simulink model "ofdm_txrx_supermimo"  (Simulink 7.1)
settings: Solver=VariableStepDiscrete  SolverMode=SingleTasking  StartTime=0.0  StopTime=simOnly_simLength  RelTol=1e-3
PostLoadFcn:
  | ofdm_rx_supermimo_init
InitFcn:
  | ofdm_rx_supermimo_init
StartFcn:
  | ofdm_rx_supermimo_init
values from neighbouring files (symbols this model uses):
  Action0_Config = 0* 31*2^18 + 0*2^24 + PHY_AUTORESPONSE_REQ_GOODHDR + PHY_AUTORESPONSE_REQ_GOODPKT + PHY_AUTORESPONSE_REQ_FLAGA    [ofdm_tx_supermimo_init.m]
  Action1_Config = 0* 62*2^18 + PHY_AUTORESPONSE_REQ_GOODHDR + PHY_AUTORESPONSE_REQ_GOODPKT    [ofdm_tx_supermimo_init.m]
  Action2_Config = 0    [ofdm_tx_supermimo_init.m]
  Action3_Config = 0    [ofdm_tx_supermimo_init.m]
  Action4_Config = 0    [ofdm_tx_supermimo_init.m]
  Action5_Config = 0    [ofdm_tx_supermimo_init.m]
  ActionID_AFTransmit = 31    [ofdm_tx_supermimo_init.m]
  ActionID_Disabled = 0    [ofdm_tx_supermimo_init.m]
  ActionID_SetFlagA = 62    [ofdm_tx_supermimo_init.m]
  ActionID_SetFlagB = 61    [ofdm_tx_supermimo_init.m]
  BER_RAM_init_size = 2048    [ofdm_tx_supermimo_init.m]
  CPLength = 16    [ofdm_tx_supermimo_init.m]
  longCorr_coef_i = [-4*real(LTS) 0]    [ofdm_rx_supermimo_init.m]
  longCorr_coef_q = [4*imag(LTS) 0]    [ofdm_rx_supermimo_init.m]
  Match0_Config = 0    [ofdm_tx_supermimo_init.m]
  Match1_Config = 0    [ofdm_tx_supermimo_init.m]
  Match2_Config = 0    [ofdm_tx_supermimo_init.m]
  Match3_Config = 0    [ofdm_tx_supermimo_init.m]
  Match4_Config = 0    [ofdm_tx_supermimo_init.m]
  Match5_Config = 0    [ofdm_tx_supermimo_init.m]
  Match6_Config = 0    [ofdm_tx_supermimo_init.m]
  Match7_Config = 0    [ofdm_tx_supermimo_init.m]
  max_CP_length = 16    [ofdm_tx_supermimo_init.m]
  max_num_baseRateSymbols = 31    [ofdm_tx_supermimo_init.m]
  max_num_subcarriers = 64    [ofdm_tx_supermimo_init.m]
  max_num_trainingSymbols = 15    [ofdm_tx_supermimo_init.m]
  max_numBytes = 16384    [ofdm_tx_supermimo_init.m]
  max_OFDM_symbols = 511    [ofdm_tx_supermimo_init.m]
  modConstellation_qam16 = .75*[1 3 -1 -3]./3    [ofdm_tx_supermimo_init.m]
  modConstellation_qam64 = 0.875*[3 1 5 7 -3 -1 -5 -7]./7    [ofdm_tx_supermimo_init.m]
  modConstellation_qpsk = [1 -1]./sqrt(2)    [ofdm_tx_supermimo_init.m]
  numBaseRateSymbols = ceil(numHeaderBytes / numBytes_BaseRateOFDMSymbol)    [ofdm_tx_supermimo_init.m]
  numSubcarriers = 64    [ofdm_tx_supermimo_init.m]
  numTrainingSymbols = 2    [ofdm_tx_supermimo_init.m]
  pilot_indicies = 7 + ( (64-21) * 2^8) + ( (64-7) * 2^16) + (21 * 2^24)    (= 356068103)    [ofdm_tx_supermimo_init.m]
  pilotValues = hex2dec('A57D') + (2^16 * hex2dec('5A82'))    [ofdm_tx_supermimo_init.m]
  pkt_crossCorr_thresh = 0.7    [ofdm_rx_supermimo_init.m]
  pkt_energy_thresh = 0    [ofdm_rx_supermimo_init.m]
  pktByteNums = numHeaderBytes +  				byteIndex_numPayloadBytes(1)*2^8 +  				byteIndex_numPayloadBytes(2)*2^16 +  				byteIndex_simpleDynModMasks * 2^24    [ofdm_rx_supermimo_init.m]
  PktDet_Delay = 58+32 +      Symbol_Timing_Offset*2^7 +      0*2^16 +      (117* 2^24)    (= 1962935642)    [ofdm_rx_supermimo_init.m]
  pktTiming_count_payloadStart = pktTiming_count_lsStart + pktTiming_ls_num*pktTiming_ls_length    [ofdm_rx_supermimo_init.m]
  preamble = preamble_scale*[shortsyms_10 longsyms_2]    [ofdm_tx_supermimo_init.m]
  preamble_ROM = preamble_I_ROM + preamble_Q_ROM*2^16    [ofdm_tx_supermimo_init.m]
  RAM_init_size = max_numBytes    (= 16384)    [ofdm_tx_supermimo_init.m]
  reg_AF_Tx_Blanking = (320+80) + ( (320+80+79)*2^16)    (= 31392144)    [ofdm_rx_supermimo_init.m]
  reg_AF_Tx_Scaling = 2944    [ofdm_rx_supermimo_init.m]
  reg_Interrupt_PktBuf_Control = reg_InterruptControl +  	1 * 2^16 +  	0 * 2^24    (= 65656)    [ofdm_rx_supermimo_init.m]
  reg_PilotCalcParams = (4 * 2^16) + (minPilotChanMag)    (= 262184.96)    [ofdm_rx_supermimo_init.m]
  regRx_chanEst_minMag = ((0.5)*2^15) + ((0.5 * 2^15)*2^16)    (= 1073758208)    [ofdm_rx_supermimo_init.m]
  regRx_coarseCalcCorrection = round((1.3e-3 - 1.2982e-3)*2^32)    [ofdm_rx_supermimo_init.m]
  regRx_pilotCalcCorrection = round(1.00115 * 2^31)    [ofdm_rx_supermimo_init.m]
  regRx_preCFOoptions = 0 * 2^0 +  	1 * 2^1 +  	0    (= 2)    [ofdm_rx_supermimo_init.m]
  rx_controlBits = 1 * 2  		 + 1 * 4  		 + 0 * 8  		 + 1 * 16 * rx_SISO_Mode  		 + 1 * 32  		 + 1 * 64  		 + 1 * 128  		 + 1 * 256  		 + 1 * 512  		 + 1 * 1024  		 + 1 * 4096  		 + 0 * 16384  		 + 0 * 32768  		 + 1 * 65536  		 + 0 * 131072  		 + 0 * 262144  		 + 1 * 524288  		 + 1 * 1048576 * tx_Alamouti_Mode  		 + 0 * 2097152           + 1 * 33554432  		 + 0 * 4194304  		 + 0 * 8388608  		 + 0 * 16777216  		 + 1 *  ...(+49 chars)    [ofdm_rx_supermimo_init.m]
  Rx_PktDet_LongCorr_Params = LongCorrCounterLoad + 0*2^8 + 0*2^16 + 255*2^24    (= 4278190325)    [ofdm_rx_supermimo_init.m]
  Rx_PktDet_LongCorrThresholds = 8000    [ofdm_rx_supermimo_init.m]
  rx_postEq_scaling = round(rxScaling*2^11) * (1 + 2^16)    [ofdm_rx_supermimo_init.m]
  RxDataScrambling_Seq = [40 198 78 63 82 173 102 245 48 111 172 115 147 230 216 93 72 65 62 2 205 242 122 90 128 83 105 97 73 10 5 252]    [ofdm_tx_supermimo_init.m]
  RxReg_FixedPktLen = 0    [ofdm_rx_supermimo_init.m]
  shortSymbol_time = shortSymbol_time(1:16).'    [ofdm_tx_supermimo_init.m]
  simOnly_numSamples = length(preamble)+( (numSubcarriers+CPLength)*(numTrainingSymbols + numBaseRateSymbols + numFullRateSymbols/2) )    [ofdm_tx_supermimo_init.m]
  train = [train train]    [ofdm_tx_supermimo_init.m]
  tx_controlBits = (antB_preambleShift * 2^4) + (2^1 * tx_Alamouti_Mode) +  	(2^0 * tx_SISO_Mode) + 4*0 + 8*1 + 0*256 + 512*0 + 1024*0 +  	0*hex2dec('1000') + 0*hex2dec('2000') + 0*hex2dec('4000') +  	1*hex2dec('8000') + 1*hex2dec('10000') + hex2dec('20000') +  	16*hex2dec('100000')    [ofdm_tx_supermimo_init.m]
  tx_delays = (50*2^0) + (0*2^8) + (30*2^12)    (= 122930)    [ofdm_tx_supermimo_init.m]
  tx_scaling = (3072) + (12288 * 2^16)    (= 805309440)    [ofdm_tx_supermimo_init.m]
  TxDataScrambling_Seq = [40 198 78 63 82 173 102 245 48 111 172 115 147 230 216 93 72 65 62 2 205 242 122 90 128 83 105 97 73 10 5 252]    [ofdm_tx_supermimo_init.m]
  txfilt_firpm = 0.95*txfilt_firpm./(max(txfilt_firpm))    [ofdm_tx_supermimo_init.m]
  txfilt_intfilt = [0 intfilt(4,4,.8)]    [ofdm_tx_supermimo_init.m]
  txReg_symbolCounts = (2^8 *numBaseRateSymbols ) + numTrainingSymbols    [ofdm_tx_supermimo_init.m]
  TxRx_FFTScaling = (3 * 2^0) +  	(2 * 2^2) +  	(1 * 2^4) +  	(1 * 2^6) +  	(1 * 2^8) +  	(0 * 2^10)    (= 347)    [ofdm_tx_supermimo_init.m]
undefined symbols (not defined in the file or in its folder): AntA, AntB, BaseRate, Down, gcbh, numBuffs, numBytes, Up, xlBlockLoadCallback, xlGetSimulinkPeriod, xlipgetpartsetting
libraries: xbsIndex_r4 x6035, simulink x4, dspsigops x4, commchan3 x2, dspsrcs4 x2, commrflib2 x2
(part 7 of 61)

== system ofdm_txrx_supermimo/OFDM Tx MIMO/OutputMuxes ==
  note: Cheap output-enable circuit. Output registers are
      | brought out of reset on the first 0->1 transition in the
      | LSB of Pream Index. In other words, the outputs are
      | enabled when the preamble starts, and disabled when
      | the entire packet is finished.
  Pream Index : Inport  OutDataType=sfix(16)  OutScaling=2^0
  AntA I : Inport  Port=2  OutDataType=sfix(16)  OutScaling=2^0
  AntA Q : Inport  Port=3  OutDataType=sfix(16)  OutScaling=2^0
  AntB I : Inport  Port=4  OutDataType=sfix(16)  OutScaling=2^0
  AntB Q : Inport  Port=5  OutDataType=sfix(16)  OutScaling=2^0
  vin : Inport  Port=6  OutDataType=sfix(16)  OutScaling=2^0
  Pkt Done : Inport  Port=7  OutDataType=sfix(16)  OutScaling=2^0
  Antenna Swapping : Subsystem
  Constant1 : xbsIndex_r4/Constant  arith_type=Boolean  const=0  n_bits=1  bin_pt=0  explicit_period=off  period=2  equ=P=C  opselect=C  inp2=PCIN>>17  opr=+  inp1=P  carry=CIN  dbl_ovrd=off  block_type=constant
      settings: block_version=VER_STRING_GOES_HERE
  Constant7 : xbsIndex_r4/Constant  arith_type=Boolean  const=1  n_bits=1  bin_pt=0  explicit_period=off  period=2  equ=P=C  opselect=C  inp2=PCIN>>17  opr=+  inp1=P  carry=CIN  dbl_ovrd=off  block_type=constant
      settings: block_version=VER_STRING_GOES_HERE
  Delay1 : xbsIndex_r4/Delay  en=off  latency=1  dbl_ovrd=off  reg_retiming=off  block_type=delay
      settings: infoedit=Hardware notes: A delay line is a chain, each link of which is an SRL16 followed by a flip-flop. If register retiming is enabled, the delay line is a chain of flip-flops.  block_version=VER_STRING_GOES_HERE
  Delay2 : xbsIndex_r4/Delay  en=off  latency=1  dbl_ovrd=off  reg_retiming=off  block_type=delay
      settings: infoedit=Hardware notes: A delay line is a chain, each link of which is an SRL16 followed by a flip-flop. If register retiming is enabled, the delay line is a chain of flip-flops.  block_version=VER_STRING_GOES_HERE
  Inverter : xbsIndex_r4/Inverter  en=off  latency=0  dbl_ovrd=off  block_type=inv
      settings: xl_area=[0, 0, 0, 0, 0, 0, 0]  block_version=VER_STRING_GOES_HERE
  LSB : xbsIndex_r4/Slice  nbits=1  boolean_output=on  mode=Lower Bit Location + Width  bit1=0  base1=MSB of Input  bit0=0  base0=LSB of Input  dbl_ovrd=off  block_type=slice
      settings: infoedit="Extracts a given range of bits from each input sample and presents it at the output.  The output type is ordinarily unsigned with binary point at zero, but can be Boolean when the slice is one bit wide.<P><P>Hardware notes: In hardware this block costs nothing."  block_version=VER_STRING_GOES_HERE
  Logical1 : xbsIndex_r4/Logical  logical_function=OR  inputs=2  en=off  latency=0  precision=Full  arith_type=Unsigned  n_bits=8  bin_pt=2  align_bp=on  dbl_ovrd=off  block_type=logical
      settings: xl_area=[1 0 0 1 0 0 0]  block_version=VER_STRING_GOES_HERE
  Logical2 : xbsIndex_r4/Logical  logical_function=OR  inputs=2  en=off  latency=0  precision=Full  arith_type=Unsigned  n_bits=8  bin_pt=2  align_bp=on  dbl_ovrd=off  block_type=logical
      settings: xl_area=[1 0 0 1 0 0 0]  block_version=VER_STRING_GOES_HERE
  Logical3 : xbsIndex_r4/Logical  logical_function=OR  inputs=2  en=off  latency=0  precision=Full  arith_type=Unsigned  n_bits=8  bin_pt=2  align_bp=on  dbl_ovrd=off  block_type=logical
      settings: xl_area=[1 0 0 1 0 0 0]  block_version=VER_STRING_GOES_HERE
  Mux{,1..3} (4 alike) : xbsIndex_r4/Mux  inputs=2  en=off  latency=0  precision=User Defined  arith_type="Signed  (2's comp)"  n_bits=14  bin_pt=13  quantization=Truncate  overflow=Wrap  dbl_ovrd=off  block_type=mux
      settings: xl_area=[7 0 0 14 0 0 0]  block_version=VER_STRING_GOES_HERE
  Payload Running : xbsIndex_r4/Register  init=0  rst=on  en=on  dbl_ovrd=off  block_type=register
      settings: xl_area=[1 1 0 0 0 0 0]  block_version=VER_STRING_GOES_HERE
  Payload Running1 : xbsIndex_r4/Register  init=1  rst=on  en=on  dbl_ovrd=off  block_type=register
      settings: xl_area=[1 1 0 0 0 0 0]  block_version=VER_STRING_GOES_HERE
  Posedge2 : Subsystem
  Preamble Generation : Subsystem
  Preambles : Scope  NumInputPorts=7
      settings: TimeRange=9328  YMin=-0.2~-0.25~-1~-1~0~0~0  YMax=0.15~0.15~1~1~1~3500~1  SaveName=ScopeData13
  Register{,1..3} (4 alike) : xbsIndex_r4/Register  init=0  rst=on  en=off  dbl_ovrd=off  block_type=register
      settings: xl_area=[0, 0, 0, 0, 0, 0, 0]  block_version=VER_STRING_GOES_HERE
  TxDone Logic : Subsystem
  TxRunning Outputs : Subsystem
  done1 : xbsIndex_r4/Gateway Out  hdl_port=off  timing_constraint=None  locs_specified=off  LOCs={}  block_type=gatewayout
      settings: infoedit="Gateway out block.  Converts Xilinx fixed point inputs into ouputs of type Simulink integer, double, or fixed point.<P><P>Hardware notes:  In hardware these blocks become top level output ports or are discarded, depending on how they are configured."  xl_area=[0 0 0 0 1 0 0]  block_version=VER_STRING_GOES_HERE
  done4 : xbsIndex_r4/Gateway Out  hdl_port=off  timing_constraint=None  locs_specified=off  LOCs={}  block_type=gatewayout
      settings: infoedit="Gateway out block.  Converts Xilinx fixed point inputs into ouputs of type Simulink integer, double, or fixed point.<P><P>Hardware notes:  In hardware these blocks become top level output ports or are discarded, depending on how they are configured."  xl_area=[0 0 0 0 1 0 0]  block_version=VER_STRING_GOES_HERE
  done5 : xbsIndex_r4/Gateway Out  hdl_port=off  timing_constraint=None  locs_specified=off  LOCs={}  block_type=gatewayout
      settings: infoedit="Gateway out block.  Converts Xilinx fixed point inputs into ouputs of type Simulink integer, double, or fixed point.<P><P>Hardware notes:  In hardware these blocks become top level output ports or are discarded, depending on how they are configured."  xl_area=[0 0 0 0 1 0 0]  block_version=VER_STRING_GOES_HERE
  Payload : Outport  OutDataType=sfix(16)  OutScaling=2^0
  " AntA I" : Outport  Port=2  OutDataType=sfix(16)  OutScaling=2^0
  " AntA Q" : Outport  Port=3  OutDataType=sfix(16)  OutScaling=2^0
  " AntB I" : Outport  Port=4  OutDataType=sfix(16)  OutScaling=2^0
  " AntB Q" : Outport  Port=5  OutDataType=sfix(16)  OutScaling=2^0
  tag scope: Tx_Running=global
  wires:
  Logical1/1 -> Mux/1, Mux1/1, Mux2/1, Mux3/1, Logical3/1
  Constant7/1 -> Payload Running/1
  TxDone Logic/1(Done) -> Payload Running/2, Delay1/1
  vin/1 -> Payload Running/3, Logical1/2
  AntA I/1 -> Mux/3
  AntA Q/1 -> Mux1/3
  Constant1/1 -> Payload Running1/1
  Payload Running1/1 -> Antenna Swapping/1(Rst), Register/2, Register1/2, Register2/2, Register3/2, Inverter/1
  LSB/1 -> Posedge2/1(D)
  Logical2/1 -> LSB/1, Antenna Swapping/2(PreamIndex), Preamble Generation/1(SampInd)
  Preamble Generation/2(AntA_Q) -> Mux1/2
  Register/1 -> " AntA I"/1
  Register1/1 -> " AntA Q"/1
  Mux2/1 -> Antenna Swapping/5(AntB_I)
  Mux3/1 -> Antenna Swapping/6(AntB_Q)
  Register2/1 -> " AntB I"/1
  Register3/1 -> " AntB Q"/1
  AntB I/1 -> Mux2/3
  AntB Q/1 -> Mux3/3
  Preamble Generation/3(AntB_I) -> Mux2/2
  Preamble Generation/4(AntB_Q) -> Mux3/2
  Payload Running/1 -> Logical1/1
  Delay1/1 -> Payload Running1/2
  Delay2/1 -> Payload/1
  Preamble Generation/1(AntA_I) -> Mux/2, done1/1
  Mux/1 -> Antenna Swapping/3(AntA_I)
  Mux1/1 -> Antenna Swapping/4(AntA_Q)
  Antenna Swapping/2(" AntA_I") -> Register/1
  Antenna Swapping/3(" AntA_Q") -> Register1/1
  Antenna Swapping/4(" AntB_I") -> Register2/1
  Antenna Swapping/5(" AntB_Q") -> Register3/1
  Pream Index/1 -> Logical2/2, done5/1
  [AF_PreambleIndex] -> Logical2/1, done4/1
  Posedge2/1(Q) -> Payload Running1/3
  Pkt Done/1 -> TxDone Logic/1(Pkt Done)
  Logical3/1 -> Delay2/1
  Antenna Swapping/1(AF Payload) -> Logical3/2
  Inverter/1 -> [Tx_Running], TxRunning Outputs/1(Tx)
  done4/1 -> Preambles/1
  done5/1 -> Preambles/2
  done1/1 -> Preambles/3

== system ofdm_txrx_supermimo/OFDM Tx MIMO/Pre-Spin,  Filters & DACs ==
  note: Delay for mults (2)
      | and filters (6)
  Payload : Inport  OutDataType=sfix(16)  OutScaling=2^0
  A_I : Inport  Port=2  OutDataType=sfix(16)  OutScaling=2^0
  A_Q : Inport  Port=3  OutDataType=sfix(16)  OutScaling=2^0
  B_I : Inport  Port=4  OutDataType=sfix(16)  OutScaling=2^0
  B_Q : Inport  Port=5  OutDataType=sfix(16)  OutScaling=2^0
  A*conj(B) : Subsystem
  "A*conj(B) " : Subsystem
  Constant1 : xbsIndex_r4/Constant  arith_type=Signed (2's comp)  const=0  n_bits=16  bin_pt=15  explicit_period=on  period=4  equ=P=C  opselect=C  inp2=PCIN>>17  opr=+  inp1=P  carry=CIN  dbl_ovrd=off  block_type=constant
      settings: block_version=VER_STRING_GOES_HERE
  Constant2 : xbsIndex_r4/Constant  arith_type=Signed (2's comp)  const=1  n_bits=16  bin_pt=15  explicit_period=on  period=4  equ=P=C  opselect=C  inp2=PCIN>>17  opr=+  inp1=P  carry=CIN  dbl_ovrd=off  block_type=constant
      settings: block_version=VER_STRING_GOES_HERE
  Convert1 : xbsIndex_r4/Convert  arith_type=Unsigned  n_bits=1  bin_pt=0  quantization=Truncate  overflow=Wrap  latency=0  dbl_ovrd=off  pipeline=off  block_type=convert
      settings: xl_area=[0, 0, 0, 0, 0, 0, 0]  block_version=VER_STRING_GOES_HERE
  Delay1 : xbsIndex_r4/Delay  en=off  latency=8  dbl_ovrd=off  reg_retiming=off  block_type=delay
  Down Sample : xbsIndex_r4/Down Sample  sample_ratio=2  sample_phase="Last Value of Frame  (most efficient)"  en=off  latency=1  dbl_ovrd=off  block_type=dsamp
      settings: block_version=VER_STRING_GOES_HERE
  Filters : Subsystem
  Logical1 : xbsIndex_r4/Logical  logical_function=OR  inputs=2  en=off  latency=0  precision=Full  arith_type=Unsigned  n_bits=8  bin_pt=2  align_bp=on  dbl_ovrd=off  block_type=logical
      settings: xl_area=[1 0 0 1 0 0 0]  block_version=VER_STRING_GOES_HERE
  Mult{1..3,7} (4 alike) : xbsIndex_r4/Mult  precision=User Defined  arith_type="Signed  (2's comp)"  n_bits=16  bin_pt=15  quantization=Truncate  overflow=Saturate  en=off  latency=1  dbl_ovrd=off  use_behavioral_HDL=off  use_embedded=on  opt=Speed  optimum_pipeline=off  pipeline=on  use_rpm=on  placement_style=Triangular  block_type=mult
      settings: infoedit=Hardware notes: To use the internal pipeline stage of the dedicated multiplier you must select 'Pipeline for maximum performance'.  block_version=10.1.3
  Mux1 : xbsIndex_r4/Mux  inputs=2  en=off  latency=0  precision=Full  arith_type=Unsigned  n_bits=16  bin_pt=14  quantization=Truncate  overflow=Wrap  dbl_ovrd=off  block_type=mux
      settings: block_version=VER_STRING_GOES_HERE
  Mux3 : xbsIndex_r4/Mux  inputs=2  en=off  latency=0  precision=Full  arith_type=Unsigned  n_bits=16  bin_pt=14  quantization=Truncate  overflow=Wrap  dbl_ovrd=off  block_type=mux
      settings: block_version=VER_STRING_GOES_HERE
  Mux4 : xbsIndex_r4/Mux  inputs=2  en=off  latency=0  precision=Full  arith_type=Unsigned  n_bits=16  bin_pt=14  quantization=Truncate  overflow=Wrap  dbl_ovrd=off  block_type=mux
      settings: block_version=VER_STRING_GOES_HERE
  Tx : Scope  NumInputPorts=7
      settings: TimeRange=9328  YMin=-1~-1~-1~-1~-0.4~-0.4~0.25  YMax=1~1~1~1~0.4~0.5~1.5  SaveName=ScopeData9
  Tx_AntA_DAC_I : xbsIndex_r4/Gateway Out  hdl_port=on  timing_constraint=None  locs_specified=off  LOCs={}  block_type=gatewayout
      settings: infoedit="Gateway out block.  Converts Xilinx fixed point inputs into ouputs of type Simulink integer, double, or fixed point.<P><P>Hardware notes:  In hardware these blocks become top level output ports or are discarded, depending on how they are configured."  block_version=10.1.3
  Tx_AntA_DAC_Q : xbsIndex_r4/Gateway Out  hdl_port=on  timing_constraint=None  locs_specified=off  LOCs={}  block_type=gatewayout
      settings: infoedit="Gateway out block.  Converts Xilinx fixed point inputs into ouputs of type Simulink integer, double, or fixed point.<P><P>Hardware notes:  In hardware these blocks become top level output ports or are discarded, depending on how they are configured."  block_version=10.1.3
  Tx_AntB_DAC_I : xbsIndex_r4/Gateway Out  hdl_port=on  timing_constraint=None  locs_specified=off  LOCs={}  block_type=gatewayout
      settings: infoedit="Gateway out block.  Converts Xilinx fixed point inputs into ouputs of type Simulink integer, double, or fixed point.<P><P>Hardware notes:  In hardware these blocks become top level output ports or are discarded, depending on how they are configured."  block_version=10.1.3
  Tx_AntB_DAC_Q : xbsIndex_r4/Gateway Out  hdl_port=on  timing_constraint=None  locs_specified=off  LOCs={}  block_type=gatewayout
      settings: infoedit="Gateway out block.  Converts Xilinx fixed point inputs into ouputs of type Simulink integer, double, or fixed point.<P><P>Hardware notes:  In hardware these blocks become top level output ports or are discarded, depending on how they are configured."  block_version=10.1.3
  Up Sample13 : xbsIndex_r4/Up Sample  sample_ratio=2  copy_samples=on  dbl_ovrd=off  block_type=usamp
      settings: infoedit="Up samples input data.  Inserted values  can be zeros or copies of the most recent input sample.<P><P>Hardware notes: No hardware is needed if inserted values are copies of the input sample; otherwise, a mux and single bit flip-flop are used."  block_version=10.1.3
  done{1..7} (7 alike) : xbsIndex_r4/Gateway Out  hdl_port=off  timing_constraint=None  locs_specified=off  LOCs={}  block_type=gatewayout
      settings: infoedit="Gateway out block.  Converts Xilinx fixed point inputs into ouputs of type Simulink integer, double, or fixed point.<P><P>Hardware notes:  In hardware these blocks become top level output ports or are discarded, depending on how they are configured."  xl_area=[0 0 0 0 1 0 0]  block_version=VER_STRING_GOES_HERE
  " A_I" : Outport  OutDataType=sfix(16)  OutScaling=2^0
  " A_Q" : Outport  Port=2  OutDataType=sfix(16)  OutScaling=2^0
  " B_I" : Outport  Port=3  OutDataType=sfix(16)  OutScaling=2^0
  " B_Q" : Outport  Port=4  OutDataType=sfix(16)  OutScaling=2^0
  wires:
  Filters/1(" I_A") -> Mult7/2, done3/1
  Tx_AntA_DAC_I/1 -> " A_I"/1
  Filters/2(" Q_A") -> Mult1/2, done6/1
  Tx_AntA_DAC_Q/1 -> " A_Q"/1
  Tx_AntB_DAC_I/1 -> " B_I"/1
  Tx_AntB_DAC_Q/1 -> " B_Q"/1
  Filters/4(" Q_B") -> Mult3/2
  Payload/1 -> Delay1/1
  [TxReg_PreambleScaling] -> Mux4/2
  [TxReg_postIFFTScaling] -> Mux4/3
  Mult1/1 -> Tx_AntA_DAC_Q/1, done1/1
  Mux4/1 -> Mult7/1, Mult1/1, Mult2/1, Mult3/1, done7/1
  Up Sample13/1 -> Mux4/1
  Filters/3(" I_B") -> Mult2/2
  Mult3/1 -> Tx_AntB_DAC_Q/1
  Mult2/1 -> Tx_AntB_DAC_I/1
  B_Q/1 -> "A*conj(B) "/4(Im[A])
  B_I/1 -> "A*conj(B) "/3(Re[A])
  [RxAutoReplyProc_UsePreCFO] -> Convert1/1
  [CFO_DDSOut_I] -> Mux1/3
  [CFO_DDSOut_Q] -> Mux3/3
  Down Sample/1 -> Mux3/1, Mux1/1
  Constant2/1 -> Mux1/2
  Constant1/1 -> Mux3/2
  Logical1/1 -> Down Sample/1
  [regTx_AlwaysUsePreSpin] -> Logical1/1
  Convert1/1 -> Logical1/2
  Delay1/1 -> Up Sample13/1
  Mux1/1 -> A*conj(B)/4(Re[B]), "A*conj(B) "/1(Re[B])
  Mux3/1 -> A*conj(B)/3(Im[B]), "A*conj(B) "/2(Im[B])
  A_I/1 -> A*conj(B)/1(Re[A])
  A_Q/1 -> A*conj(B)/2(Im[A])
  Mult7/1 -> Tx_AntA_DAC_I/1, done2/1
  done4/1 -> Tx/1
  done5/1 -> Tx/2
  done3/1 -> Tx/3
  done6/1 -> Tx/4
  done2/1 -> Tx/5
  done1/1 -> Tx/6
  done7/1 -> Tx/7
  A*conj(B)/1(Re) -> Filters/1(I_A), done4/1
  A*conj(B)/2(Im) -> Filters/2(Q_A), done5/1
  "A*conj(B) "/1(Re) -> Filters/3(I_B)
  "A*conj(B) "/2(Im) -> Filters/4(Q_B)

== system ofdm_txrx_supermimo/OFDM Tx MIMO/Training_Data ==
  CPn_Data : Inport  OutDataType=sfix(16)  OutScaling=2^0
  train_pay : Inport  Port=2  OutDataType=sfix(16)  OutScaling=2^0
  BaseRate_En : Inport  Port=3  OutDataType=sfix(16)  OutScaling=2^0
  FFT En : Inport  Port=4  OutDataType=sfix(16)  OutScaling=2^0
  Start : Inport  Port=5  OutDataType=sfix(16)  OutScaling=2^0
  packet_done : Inport  Port=6  OutDataType=sfix(16)  OutScaling=2^0
  xn_index : Inport  Port=7  OutDataType=sfix(16)  OutScaling=2^0
  Ant Selector : Subsystem
  Convert2 : xbsIndex_r4/Convert  arith_type="Signed  (2's comp)"  n_bits=16  bin_pt=15  quantization=Truncate  overflow=Wrap  latency=0  dbl_ovrd=off  pipeline=off  block_type=convert
      settings: xl_area=[0 0 0 0 0 0 0]  block_version=VER_STRING_GOES_HERE
  Convert3 : xbsIndex_r4/Convert  arith_type="Signed  (2's comp)"  n_bits=16  bin_pt=15  quantization=Truncate  overflow=Wrap  latency=0  dbl_ovrd=off  pipeline=off  block_type=convert
      settings: xl_area=[0 0 0 0 0 0 0]  block_version=VER_STRING_GOES_HERE
  Delay2 : xbsIndex_r4/Delay  en=off  latency=1  dbl_ovrd=off  reg_retiming=off  block_type=delay
      settings: infoedit=Hardware notes: A delay line is a chain, each link of which is an SRL16 followed by a flip-flop. If register retiming is enabled, the delay line is a chain of flip-flops.  xl_area=[1 1 0 0 0 0 0]  block_version=VER_STRING_GOES_HERE
  FlexibleMod : Subsystem
  Logical : xbsIndex_r4/Logical  logical_function=AND  inputs=3  en=off  latency=0  precision=Full  arith_type=Unsigned  n_bits=8  bin_pt=2  align_bp=on  dbl_ovrd=off  block_type=logical
      settings: xl_area=[0, 0, 0, 0, 0, 0, 0]  block_version=VER_STRING_GOES_HERE
  Logical1 : xbsIndex_r4/Logical  logical_function=OR  inputs=2  en=off  latency=0  precision=Full  arith_type=Unsigned  n_bits=8  bin_pt=2  align_bp=on  dbl_ovrd=off  block_type=logical
      settings: xl_area=[0, 0, 0, 0, 0, 0, 0]  block_version=VER_STRING_GOES_HERE
  Logical5 : xbsIndex_r4/Logical  logical_function=AND  inputs=2  en=off  latency=0  precision=Full  arith_type=Unsigned  n_bits=8  bin_pt=2  align_bp=on  dbl_ovrd=off  block_type=logical
      settings: xl_area=[0, 0, 0, 0, 0, 0, 0]  block_version=VER_STRING_GOES_HERE
  Training_Pilots : Subsystem
  tx_Q : Outport  OutDataType=sfix(16)  OutScaling=2^0
  tx_I : Outport  Port=2  OutDataType=sfix(16)  OutScaling=2^0
  Payload Done : Outport  Port=3  OutDataType=sfix(16)  OutScaling=2^0
  wires:
  FlexibleMod/2(Q) -> Training_Pilots/3(Q_mod)
  FlexibleMod/1(I) -> Training_Pilots/2(I_mod)
  packet_done/1 -> FlexibleMod/2(packet_done), Logical1/1
  xn_index/1 -> FlexibleMod/1(ifft_index), Training_Pilots/1(Xn_index)
  train_pay/1 -> Delay2/1
  Logical/1 -> FlexibleMod/3(vin)
  Start/1 -> FlexibleMod/4(start)
  BaseRate_En/1 -> Ant Selector/1(BaseRate), Logical5/2
  CPn_Data/1 -> Ant Selector/2(CP#_Data), Logical/2
  Delay2/1 -> Logical5/1, Training_Pilots/4(train#), Logical/3
  Logical5/1 -> FlexibleMod/5(BaseRate_En)
  Ant Selector/1(Sel) -> FlexibleMod/6(ant_sel), Training_Pilots/5(antSel)
  FFT En/1 -> Logical/1
  Training_Pilots/1(tx_I) -> Convert3/1
  Training_Pilots/2(tx_Q) -> Convert2/1
  Convert3/1 -> tx_I/1
  Convert2/1 -> tx_Q/1
  Logical1/1 -> Training_Pilots/6(rst), Ant Selector/3(Rst)
  [TxReset] -> Logical1/2
  FlexibleMod/3(Payload Done) -> Payload Done/1

== system ofdm_txrx_supermimo/OFDM Tx MIMO/Tx Reset Logic ==
  Constant3 : Constant  Value=0  OutDataType=sfix(16)  OutScaling=2^0
  Logical : xbsIndex_r4/Logical  logical_function=OR  inputs=2  en=off  latency=0  precision=Full  arith_type=Unsigned  n_bits=16  bin_pt=0  align_bp=on  dbl_ovrd=off  block_type=logical
      settings: block_version=VER_STRING_GOES_HERE
  Pulse Generator1 : DiscretePulseGenerator  Period=1e6  PulseWidth=4  PhaseDelay=2750
  ToBool : xbsIndex_r4/Convert  arith_type=Boolean  n_bits=16  bin_pt=14  quantization=Truncate  overflow=Wrap  latency=0  dbl_ovrd=off  pipeline=off  block_type=convert
      settings: block_version=VER_STRING_GOES_HERE
  Tx_Reset : xbsIndex_r4/Gateway In  arith_type=Unsigned  n_bits=1  bin_pt=0  quantization="Round  (unbiased: +/- Inf)"  overflow=Saturate  period=4  dbl_ovrd=off  timing_constraint=None  locs_specified=off  LOCs={}  block_type=gatewayin
      settings: infoedit="Gateway in block.  Converts inputs of type Simulink integer, double and fixed point to  Xilinx fixed point type.<P><P>Hardware notes:  In hardware these blocks become top level input ports."  xl_area=[0, 0, 0, 0, 0, 0, 0]  block_version=VER_STRING_GOES_HERE
  tag scope: TxReset=global
  wires:
  Tx_Reset/1 -> Logical/1
  Logical/1 -> ToBool/1
  ToBool/1 -> [TxReset]
  Constant3/1 -> Tx_Reset/1
  [TxReg_TxReset] -> Logical/2

== system ofdm_txrx_supermimo/OFDM Tx MIMO/TxControl ==
  note: 0=Waiting for Cyclic Prefix
      | 1=Processing real data
  note: 0=Sending training symbols
      | 1=Processing real data
  note: 1 cycle delay to align CPn_data
      | with IFFT start. FFT core begins
      | accepting data 1 cycle after
      | rising edge of start.
  note: Three inputs to AND for IFFT start:
      | 1) Start when first data sample is ready
      | 2) Start when samp/sym counters are enabled
      | (i.e. during payload, not training)
      | 3) Not after last symbol is finished
      | (i.e. when coutners are being reset)
  StartTx : Inport  OutDataType=sfix(16)  OutScaling=2^0
  PktDone : Inport  Port=2  OutDataType=sfix(16)  OutScaling=2^0
  16_8LSB : xbsIndex_r4/Slice  nbits=ceil(log2(max_num_baseRateSymbols+1))  boolean_output=off  mode=Lower Bit Location + Width  bit1=0  base1=MSB of Input  bit0=8  base0=LSB of Input  dbl_ovrd=off  block_type=slice
      settings: infoedit="Extracts a given range of bits from each input sample and presents it at the output.  The output type is ordinarily unsigned with binary point at zero, but can be Boolean when the slice is one bit wide.<P><P>Hardware notes: In hardware this block costs nothing."  block_version=VER_STRING_GOES_HERE
  2x : Subsystem
  2x1 : Subsystem
  8LSB : xbsIndex_r4/Slice  nbits=ceil(log2(max_num_trainingSymbols+1))  boolean_output=off  mode=Lower Bit Location + Width  bit1=0  base1=MSB of Input  bit0=0  base0=LSB of Input  dbl_ovrd=off  block_type=slice
      settings: infoedit="Extracts a given range of bits from each input sample and presents it at the output.  The output type is ordinarily unsigned with binary point at zero, but can be Boolean when the slice is one bit wide.<P><P>Hardware notes: In hardware this block costs nothing."  block_version=VER_STRING_GOES_HERE
  AddSub1 : xbsIndex_r4/AddSub  mode=Addition  use_carryin=off  use_carryout=off  en=off  latency=0  precision=Full  arith_type=Unsigned  n_bits=10  bin_pt=0  quantization=Truncate  overflow=Wrap  dbl_ovrd=off  use_behavioral_HDL=on  pipelined=off  use_rpm=on  hw_selection=Fabric  block_type=addsub
      settings: xl_area=[0, 0, 0, 0, 0, 0, 0]  block_version=VER_STRING_GOES_HERE
  Constant1 : xbsIndex_r4/Constant  Orientation=left  arith_type=Unsigned  const=length(preamble)-148  n_bits=ceil(log2(length(preamble)))  bin_pt=0  explicit_period=off  period=1  equ=P=C  opselect=C  inp2=PCIN>>17  opr=+  inp1=P  carry=CIN  dbl_ovrd=off  block_type=constant
      settings: block_version=VER_STRING_GOES_HERE
  Constant2 : xbsIndex_r4/Constant  arith_type=Boolean  const=1  n_bits=1  bin_pt=0  explicit_period=off  period=1  equ=P=C  opselect=C  inp2=PCIN>>17  opr=+  inp1=P  carry=CIN  dbl_ovrd=off  block_type=constant
      settings: block_version=VER_STRING_GOES_HERE
  Constant3 : xbsIndex_r4/Constant  Orientation=left  arith_type=Unsigned  const=length(preamble)-1  n_bits=ceil(log2(length(preamble)))  bin_pt=0  explicit_period=off  period=1  equ=P=C  opselect=C  inp2=PCIN>>17  opr=+  inp1=P  carry=CIN  dbl_ovrd=off  block_type=constant
      settings: block_version=VER_STRING_GOES_HERE
  Constant4 : xbsIndex_r4/Constant  Orientation=left  arith_type=Unsigned  const=numSubcarriers+CPLength-1  n_bits=ceil(log2(max_num_subcarriers+CPLength+1))  bin_pt=0  explicit_period=off  period=1  equ=P=C  opselect=C  inp2=PCIN>>17  opr=+  inp1=P  carry=CIN  dbl_ovrd=off  block_type=constant
      settings: block_version=VER_STRING_GOES_HERE
  Constant5 : xbsIndex_r4/Constant  arith_type=Boolean  const=1  n_bits=1  bin_pt=0  explicit_period=off  period=1  equ=P=C  opselect=C  inp2=PCIN>>17  opr=+  inp1=P  carry=CIN  dbl_ovrd=off  block_type=constant
      settings: block_version=VER_STRING_GOES_HERE
  Constant6 : xbsIndex_r4/Constant  arith_type=Boolean  const=1  n_bits=1  bin_pt=0  explicit_period=off  period=1  equ=P=C  opselect=C  inp2=PCIN>>17  opr=+  inp1=P  carry=CIN  dbl_ovrd=off  block_type=constant
      settings: AttributesFormatString=%<explicit_period>  block_version=VER_STRING_GOES_HERE
  Constant7 : xbsIndex_r4/Constant  arith_type=Unsigned  const=numSubcarriers-1  n_bits=log2(numSubcarriers)  bin_pt=0  explicit_period=off  period=1  equ=P=C  opselect=C  inp2=PCIN>>17  opr=+  inp1=P  carry=CIN  dbl_ovrd=off  block_type=constant
      settings: block_version=VER_STRING_GOES_HERE
  Constant8 : xbsIndex_r4/Constant  Orientation=left  arith_type=Unsigned  const=0  n_bits=1  bin_pt=0  explicit_period=off  period=1  equ=P=C  opselect=C  inp2=PCIN>>17  opr=+  inp1=P  carry=CIN  dbl_ovrd=off  block_type=constant
      settings: block_version=VER_STRING_GOES_HERE
  Convert : xbsIndex_r4/Convert  arith_type=Boolean  n_bits=8  bin_pt=6  quantization=Truncate  overflow=Wrap  latency=0  dbl_ovrd=off  pipeline=off  block_type=convert
      settings: xl_area=[0, 0, 0, 0, 0, 0, 0]  block_version=VER_STRING_GOES_HERE
  Delay : xbsIndex_r4/Delay  en=off  latency=1  dbl_ovrd=off  reg_retiming=off  block_type=delay
      settings: infoedit=Hardware notes: A delay line is a chain, each link of which is an SRL16 followed by a flip-flop. If register retiming is enabled, the delay line is a chain of flip-flops.  xl_area=[1 1 0 0 0 0 0]  block_version=VER_STRING_GOES_HERE
  Delay1 : xbsIndex_r4/Delay  en=off  latency=1  dbl_ovrd=off  reg_retiming=off  block_type=delay
      settings: infoedit=Hardware notes: A delay line is a chain, each link of which is an SRL16 followed by a flip-flop. If register retiming is enabled, the delay line is a chain of flip-flops.  xl_area=[1 1 0 0 0 0 0]  block_version=VER_STRING_GOES_HERE
  Down Sample : xbsIndex_r4/Down Sample  sample_ratio=2  sample_phase=First Value of Frame  en=off  latency=0  dbl_ovrd=off  block_type=dsamp
      settings: block_version=VER_STRING_GOES_HERE
  Down Sample1 : xbsIndex_r4/Down Sample  sample_ratio=2  sample_phase=First Value of Frame  en=off  latency=0  dbl_ovrd=off  block_type=dsamp
      settings: block_version=VER_STRING_GOES_HERE
  Down Sample4 : xbsIndex_r4/Down Sample  sample_ratio=2  sample_phase="Last Value of Frame  (most efficient)"  en=off  latency=1  dbl_ovrd=off  block_type=dsamp
      settings: block_version=VER_STRING_GOES_HERE
  FFT Start Gen : Subsystem  Orientation=left
  Logical1 : xbsIndex_r4/Logical  logical_function=OR  inputs=2  en=off  latency=0  precision=Full  arith_type=Unsigned  n_bits=8  bin_pt=2  align_bp=on  dbl_ovrd=off  block_type=logical
      settings: xl_area=[1 0 0 1 0 0 0]  block_version=VER_STRING_GOES_HERE
  Logical2 : xbsIndex_r4/Logical  logical_function=OR  inputs=3  en=off  latency=0  precision=Full  arith_type=Unsigned  n_bits=8  bin_pt=2  align_bp=on  dbl_ovrd=off  block_type=logical
      settings: xl_area=[1 0 0 1 0 0 0]  block_version=VER_STRING_GOES_HERE
  Logical4 : xbsIndex_r4/Logical  logical_function=OR  inputs=2  en=off  latency=0  precision=Full  arith_type=Unsigned  n_bits=8  bin_pt=2  align_bp=on  dbl_ovrd=off  block_type=logical
      settings: xl_area=[1 0 0 1 0 0 0]  block_version=VER_STRING_GOES_HERE
  Logical6 : xbsIndex_r4/Logical  logical_function=AND  inputs=3  en=off  latency=0  precision=Full  arith_type=Unsigned  n_bits=1  bin_pt=0  align_bp=on  dbl_ovrd=off  block_type=logical
      settings: xl_area=[1 0 0 1 0 0 0]  block_version=VER_STRING_GOES_HERE
  Logical7 : xbsIndex_r4/Logical  logical_function=OR  inputs=2  en=off  latency=0  precision=Full  arith_type=Unsigned  n_bits=8  bin_pt=2  align_bp=on  dbl_ovrd=off  block_type=logical
      settings: xl_area=[1 0 0 1 0 0 0]  block_version=VER_STRING_GOES_HERE
  Payload Running1 : xbsIndex_r4/Register  init=0  rst=on  en=on  dbl_ovrd=off  block_type=register
      settings: xl_area=[1 1 0 0 0 0 0]  block_version=VER_STRING_GOES_HERE
  Payload Running2 : xbsIndex_r4/Register  init=0  rst=on  en=on  dbl_ovrd=off  block_type=register
      settings: xl_area=[1 1 0 0 0 0 0]  block_version=VER_STRING_GOES_HERE
  Payload Running4 : xbsIndex_r4/Register  init=0  rst=on  en=on  dbl_ovrd=off  block_type=register
      settings: xl_area=[1 1 0 0 0 0 0]  block_version=VER_STRING_GOES_HERE
  Posedge1 : Subsystem
  Posedge2 : Subsystem
  Preamble Index Counter : xbsIndex_r4/Counter  cnt_type=Free Running  cnt_to=Inf  operation=Up  start_count=0  cnt_by_val=1  arith_type=Unsigned  n_bits=ceil(log2(1+length(preamble)))  bin_pt=0  load_pin=off  rst=on  en=on  explicit_period=off  period=1  dbl_ovrd=off  use_behavioral_HDL=off  use_rpm=on  implementation=Fabric  block_type=counter
      settings: xl_area=[5 9 0 9 0 0 0]  block_version=VER_STRING_GOES_HERE
  Relational{1,3,5,7} (4 alike) : xbsIndex_r4/Relational  Orientation=left  mode=a=b  en=off  latency=0  dbl_ovrd=off  block_type=relational
      Relational1 settings: xl_area=[3 0 0 5 0 0 0]  block_version=VER_STRING_GOES_HERE
      Relational3 settings: xl_area=[2 0 0 4 0 0 0]  block_version=VER_STRING_GOES_HERE
      Relational5 settings: xl_area=[3 0 0 5 0 0 0]  block_version=VER_STRING_GOES_HERE
      Relational7 settings: xl_area=[2 0 0 4 0 0 0]  block_version=VER_STRING_GOES_HERE
  Relational4 : xbsIndex_r4/Relational  mode=a<=b  en=off  latency=0  dbl_ovrd=off  block_type=relational
      settings: xl_area=[2 0 0 4 0 0 0]  block_version=VER_STRING_GOES_HERE
  Relational6 : xbsIndex_r4/Relational  mode=a<=b  en=off  latency=0  dbl_ovrd=off  block_type=relational
      settings: xl_area=[2 0 0 4 0 0 0]  block_version=VER_STRING_GOES_HERE
  Relational8 : xbsIndex_r4/Relational  mode=a>b  en=off  latency=0  dbl_ovrd=off  block_type=relational
      settings: xl_area=[2 0 0 4 0 0 0]  block_version=VER_STRING_GOES_HERE
  Sample Counter : xbsIndex_r4/Counter  cnt_type=Free Running  cnt_to=Inf  operation=Up  start_count=0  cnt_by_val=1  arith_type=Unsigned  n_bits=1+ceil(log2(numSubcarriers + CPLength + 1))  bin_pt=0  load_pin=off  rst=on  en=on  explicit_period=off  period=1  dbl_ovrd=off  use_behavioral_HDL=off  use_rpm=on  implementation=Fabric  block_type=counter
      settings: xl_area=[4 7 0 7 0 0 0]  block_version=VER_STRING_GOES_HERE
  Start/Done Control : Subsystem
  Sym Counter : xbsIndex_r4/Counter  cnt_type=Free Running  cnt_to=Inf  operation=Up  start_count=0  cnt_by_val=1  arith_type=Unsigned  n_bits=ceil(log2(max_OFDM_symbols+1))  bin_pt=0  load_pin=off  rst=on  en=on  explicit_period=off  period=1  dbl_ovrd=off  use_behavioral_HDL=off  use_rpm=on  implementation=Fabric  block_type=counter
      settings: xl_area=[4 7 0 7 0 0 0]  block_version=VER_STRING_GOES_HERE
  Up Sample : xbsIndex_r4/Up Sample  sample_ratio=2  copy_samples=on  dbl_ovrd=off  block_type=usamp
      settings: infoedit="Up samples input data.  Inserted values  can be zeros or copies of the most recent input sample.<P><P>Hardware notes: No hardware is needed if inserted values are copies of the input sample; otherwise, a mux and single bit flip-flop are used."  block_version=VER_STRING_GOES_HERE
  Preamble Index : Outport  OutDataType=sfix(16)  OutScaling=2^0
  Start : Outport  Port=2  OutDataType=sfix(16)  OutScaling=2^0
  FFT En : Outport  Port=3  OutDataType=sfix(16)  OutScaling=2^0
  BaseRate_En : Outport  Port=4  OutDataType=sfix(16)  OutScaling=2^0
  Training_Data : Outport  Port=5  OutDataType=sfix(16)  OutScaling=2^0
  CPn_Data : Outport  Port=6  OutDataType=sfix(16)  OutScaling=2^0
  Start IFFT : Outport  Port=7  OutDataType=sfix(16)  OutScaling=2^0
  tag scope: reg_numBaseRateSyms=global, reg_numTrainingSyms=global
  wires:
  Payload Running1/1 -> Down Sample1/1
  Constant2/1 -> Payload Running1/1
  Logical1/1 -> Payload Running1/2, Down Sample/1
  [localReset] -> Logical1/2
  Preamble Index Counter/1 -> Up Sample/1, Preamble Index/1
  Relational1/1 -> Logical1/1
  Constant3/1 -> Relational1/1
  Posedge1/1(Q) -> Payload Running1/3
  Sample Counter/1 -> Relational4/1, Relational3/1, Relational7/1
  Relational3/1 -> Sym Counter/2, Logical4/1
  Constant6/1 -> Payload Running4/1
  [localReset] -> Payload Running4/2
  Payload Running4/1 -> Sample Counter/2, Logical6/2
  Logical4/1 -> Sample Counter/1
  [localReset] -> Logical4/2
  [localReset] -> Sym Counter/1
  Sym Counter/1 -> Relational8/2, Relational6/2, FFT Start Gen/1(SymNum)
  Relational4/1 -> Delay1/1
  Relational6/1 -> Payload Running2/3, Logical7/2
  Posedge2/1(Q) -> Delay/1, Logical2/2
  Logical2/1 -> [localReset]
  Delay/1 -> Posedge1/1(D)
  Constant1/1 -> Relational5/2
  Relational5/1 -> Payload Running4/3
  [TxReset] -> Logical2/1
  Constant7/1 -> Relational4/2
  PktDone/1 -> Start/Done Control/1(TxDone), Logical2/3
  Constant5/1 -> Payload Running2/1
  [localReset] -> Payload Running2/2
  Payload Running2/1 -> Logical7/1
  Constant8/1 -> Relational7/2
  Relational7/1 -> Logical6/1
  Logical6/1 -> Convert/1
  Convert/1 -> Start IFFT/1
  Logical7/1 -> Training_Data/1
  Delay1/1 -> CPn_Data/1
  Relational8/1 -> BaseRate_En/1
  AddSub1/1 -> Relational8/1
  Constant4/1 -> Relational3/2
  FFT Start Gen/1(En) -> FFT En/1, Logical6/3
  2x1/1(O) -> Relational6/1, AddSub1/2, [reg_numTrainingSyms]
  2x/1(O) -> AddSub1/1, [reg_numBaseRateSyms]
  Down Sample/1 -> Preamble Index Counter/1
  Down Sample1/1 -> Preamble Index Counter/2
  Up Sample/1 -> Relational5/1, Relational1/2
  16_8LSB/1 -> 2x/1(I)
  8LSB/1 -> 2x1/1(I)
  [TxReg_OFDM_SymCounts] -> Down Sample4/1
  Down Sample4/1 -> 8LSB/1, 16_8LSB/1
  Start/Done Control/1(TxStart) -> Posedge2/1(D), Start/1
  StartTx/1 -> Start/Done Control/2(Start)

== system ofdm_txrx_supermimo/OFDM Tx MIMO/TxStart ==
  note: If TxReg_SoftTxStart_TxEn is set, the PHY
      | will assert the radio controller's TxEn port. This
      | starts the Tx state machine, which will eventually
      | assert the PHY's Tx_StartTransmit input.
      | Otherwise, the TxStart register can start the PHY
      | directly, independent of the radio controller.
  note: BufNum=31 (or X?) falls before TxEn!
  Add : Sum  OutDataType=sfix(16)  OutScaling=2^-10
  Constant1 : xbsIndex_r4/Constant  arith_type=Unsigned  const=ActionID_AFTransmit  n_bits=ceil(log2(ActionID_AFTransmit))  bin_pt=0  explicit_period=off  period=1  equ=P=C  opselect=C  inp2=PCIN>>17  opr=+  inp1=P  carry=CIN  dbl_ovrd=off  block_type=constant
  Convert : xbsIndex_r4/Convert  arith_type=Boolean  n_bits=16  bin_pt=14  quantization=Truncate  overflow=Wrap  latency=0  dbl_ovrd=off  pipeline=off  block_type=convert
      settings: block_version=10.1.3
  Copy_Downsample : Subsystem
  Inverter : xbsIndex_r4/Inverter  en=off  latency=0  dbl_ovrd=off  block_type=inv
  Inverter1 : xbsIndex_r4/Inverter  en=off  latency=0  dbl_ovrd=off  block_type=inv
      settings: xl_area=[0, 0, 0, 0, 0, 0, 0]  block_version=VER_STRING_GOES_HERE
  Logical : xbsIndex_r4/Logical  logical_function=OR  inputs=2  en=off  latency=0  precision=Full  arith_type=Unsigned  n_bits=16  bin_pt=0  align_bp=on  dbl_ovrd=off  block_type=logical
      settings: block_version=VER_STRING_GOES_HERE
  Logical1 : xbsIndex_r4/Logical  logical_function=AND  inputs=2  en=off  latency=0  precision=Full  arith_type=Unsigned  n_bits=16  bin_pt=0  align_bp=on  dbl_ovrd=off  block_type=logical
      settings: block_version=VER_STRING_GOES_HERE
  Logical3 : xbsIndex_r4/Logical  logical_function=AND  inputs=2  en=off  latency=0  precision=Full  arith_type=Unsigned  n_bits=16  bin_pt=0  align_bp=on  dbl_ovrd=off  block_type=logical
      settings: block_version=VER_STRING_GOES_HERE
  Logical4 : xbsIndex_r4/Logical  logical_function=AND  inputs=2  en=off  latency=0  precision=Full  arith_type=Unsigned  n_bits=16  bin_pt=0  align_bp=on  dbl_ovrd=off  block_type=logical
      settings: block_version=VER_STRING_GOES_HERE
  Pulse Generator1 : DiscretePulseGenerator  Period=(simOnly_numSamples*2 + 1250)*2  PhaseDelay=5
  Relational1 : xbsIndex_r4/Relational  mode=a=b  en=off  latency=0  dbl_ovrd=off  block_type=relational
      settings: block_version=10.1.3
  Tx_StartTransmit : xbsIndex_r4/Gateway In  arith_type=Boolean  n_bits=1  bin_pt=0  quantization="Round  (unbiased: +/- Inf)"  overflow=Saturate  period=1  dbl_ovrd=off  timing_constraint=None  locs_specified=off  LOCs={}  block_type=gatewayin
      settings: infoedit="Gateway in block.  Converts inputs of type Simulink integer, double and fixed point to  Xilinx fixed point type.<P><P>Hardware notes:  In hardware these blocks become top level input ports."  xl_area=[0, 0, 0, 0, 0, 0, 0]  block_version=VER_STRING_GOES_HERE
  posedge1 : Subsystem
  Start : Outport  OutDataType=sfix(16)  OutScaling=2^0
  tag scope: AF_TxStart=global
  wires:
  Tx_StartTransmit/1 -> Copy_Downsample/1(D)
  Logical/1 -> Logical3/2
  [TxReg_TxStart] -> Logical1/1
  Copy_Downsample/1(Q) -> posedge1/1(D)
  [TxReg_SoftTxStart_TxEn] -> Inverter/1
  Inverter/1 -> Convert/1
  Logical1/1 -> Logical/2
  Convert/1 -> Logical1/2
  [RxAutoReply_Action_BufNum] -> Relational1/1
  Constant1/1 -> Relational1/2
  Inverter1/1 -> Logical3/1
  Relational1/1 -> Logical4/1, Inverter1/1
  Logical3/1 -> Start/1
  Logical4/1 -> [AF_TxStart]
  posedge1/1(Q) -> Logical/1, Logical4/2
  Pulse Generator1/1 -> Add/1
  [TxEn_Output_dbl] -> Add/2
  Add/1 -> Tx_StartTransmit/1

== system ofdm_txrx_supermimo/Pkt Buffer BRAM Interface/Addr Select ==
  note: Simulation of FlexBER mode requires overriding the address selection
      | to ignore the Tx blocks, even when transmitting. This is *not* required in
      | hardware, as Tx/Rx are half duplex at a given node.
  note: Let the Rx logic control the BRAM address unless we're transmitting.
      | This check handles a very rare bug, when an incoming packet starts
      | immediately before the MAC begins a transmission.
  Constant2 : xbsIndex_r4/Constant  arith_type=Boolean  const=0  n_bits=1  bin_pt=0  explicit_period=off  period=1  equ=P=C  opselect=C  inp2=PCIN>>17  opr=+  inp1=P  carry=CIN  dbl_ovrd=off  block_type=constant
      settings: block_version=8.2.02
  Constant3 : xbsIndex_r4/Constant  arith_type=Boolean  const=1  n_bits=1  bin_pt=0  explicit_period=off  period=1  equ=P=C  opselect=C  inp2=PCIN>>17  opr=+  inp1=P  carry=CIN  dbl_ovrd=off  block_type=constant
      settings: block_version=8.2.02
  Convert{1..2,7..8} (4 alike) : xbsIndex_r4/Convert  arith_type=Unsigned  n_bits=1  bin_pt=0  quantization=Truncate  overflow=Wrap  latency=0  dbl_ovrd=off  pipeline=off  block_type=convert
      settings: block_version=10.1.3
  Inverter : xbsIndex_r4/Inverter  en=off  latency=0  dbl_ovrd=off  block_type=inv
  Inverter1 : xbsIndex_r4/Inverter  en=off  latency=0  dbl_ovrd=off  block_type=inv
  Logical : xbsIndex_r4/Logical  logical_function=OR  inputs=2  en=off  latency=0  precision=Full  arith_type=Unsigned  n_bits=16  bin_pt=0  align_bp=on  dbl_ovrd=off  block_type=logical
  Logical1 : xbsIndex_r4/Logical  logical_function=AND  inputs=2  en=off  latency=0  precision=Full  arith_type=Unsigned  n_bits=16  bin_pt=0  align_bp=on  dbl_ovrd=off  block_type=logical
  Logical2 : xbsIndex_r4/Logical  logical_function=AND  inputs=3  en=off  latency=0  precision=Full  arith_type=Unsigned  n_bits=16  bin_pt=0  align_bp=on  dbl_ovrd=off  block_type=logical
  Simulation Multiplexer1 : xbsIndex_r4/Simulation Multiplexer  sim_sel=1  hw_sel=2  block_type=simmux
      settings: infoedit="Distinguish input subsystems as \"simulation only\" and \"generation only\".  The input specified For Simulation will be used during Simulink simulation.  The input specified For Generation will be used during code generation.  This block will typically be used on the output of a HW Co-Simulation, Black Box, or ModelSim subsystem.<P><P>Hardware Notes: This block costs nothing."  block_version=10.1.3
  Sel : Outport
  wires:
  Logical2/1 -> Logical/2
  Constant2/1 -> Simulation Multiplexer1/1
  Logical1/1 -> Convert2/1
  Simulation Multiplexer1/1 -> Logical1/2
  [regRx_FlexBERMode] -> Logical1/1
  Constant3/1 -> Simulation Multiplexer1/2
  Logical/1 -> Sel/1
  [Tx_Running] -> Inverter/1
  Inverter/1 -> Convert7/1
  [BRAM_Rx_AnyWrEn] -> Convert1/1
  Convert2/1 -> Logical/1
  [regTx_PktRunning] -> Inverter1/1
  Inverter1/1 -> Convert8/1
  Convert1/1 -> Logical2/1
  Convert7/1 -> Logical2/2
  Convert8/1 -> Logical2/3
